simulink model "SdbTxSigProc"  (R2022b)
settings: Solver=FixedStepDiscrete  StartTime=0.0  StopTime=10.0  FixedStep=0.1  RelTol=1e-3
(part 1 of 4)
settings Model: Creator=blue.ge  LastModifiedBy=blue.ge
settings DataIOCC: SaveFormat=StructureWithTime
settings OptimizationCC: DefaultParameterBehavior=Inlined  EnhancedBackFolding=on  PassReuseOutputArgsAs=Individual arguments  ZeroExternalMemoryAtStartup=off  ZeroInternalMemoryAtStartup=off  OptimizeBlockOrder=speed  DifferentSizesBufferReuse=on  OptimizationCustomize=off  EfficientTunableParamExpr=on
settings DebuggingCC: ParameterTunabilityLossMsg=error
settings ModelReferenceCC: ModelReferenceNumInstancesAllowed=Single
settings SFSimCC: MATLABDynamicMemAlloc=off
settings RTWCC: Description=AUTOSAR  SystemTargetFile=autosar.tlc  GenCodeOnly=on  TemplateMakefile=ert_default_tmf
settings CodeAppCC: ForceParamTrailComments=on  IgnoreCustomStorageClasses=off  OperatorAnnotations=on  SimulinkDataObjDesc=on  SFDataObjDesc=on  InsertBlockDesc=on
settings STFCustomTargetCC: TargetLangStandard=C99 (ISO)  MultiwordLength=256  ParMdlRefBuildCompliant=on  CompOptLevelCompliant=on  IncludeMdlTerminateFcn=off  CombineOutputUpdateFcns=on  SuppressErrorStatus=on  ERTCustomFileBanners=on  SupportNonFinite=off  SupportComplex=off  SupportContinuousTime=off  SupportNonInlinedSFcns=off  RemoveResetFunc=on  MATLABClassNameForMDSCustomization=Simulink.SoftwareTarget.ERTCustomization  AutosarCompliant=on  UseToolchainInfoCompliant=on  LookupTableObjectStructAxisOrder=2,1,3,4,...  LUTObjectStructOrderEvenSpacing=Size,Table,Breakpoints  SystemTargetFile=autosar.tlc
settings ERTTargetCC: TargetLangStandard=C99 (ISO)  ConcurrentExecutionCompliant=off  IncludeMdlTerminateFcn=off  SuppressErrorStatus=on  SupportNonFinite=off  SupportComplex=off  ModelStepFunctionPrototypeControlCompliant=off  CPPClassGenCompliant=off  AutosarCompliant=on  LookupTableObjectStructAxisOrder=2,1,3,4,...  LUTObjectStructOrderEvenSpacing=Size,Table,Breakpoints  InlinedParameterPlacement=NonHierarchical  ERTCustomFileTemplate=example_file_process.tlc

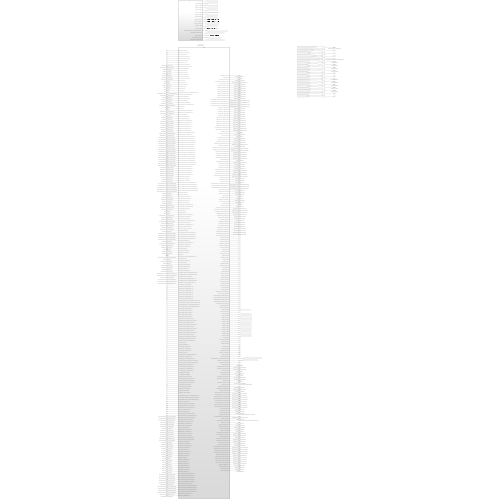
[thumbnail: Simulink's stored preview of the top-level diagram]
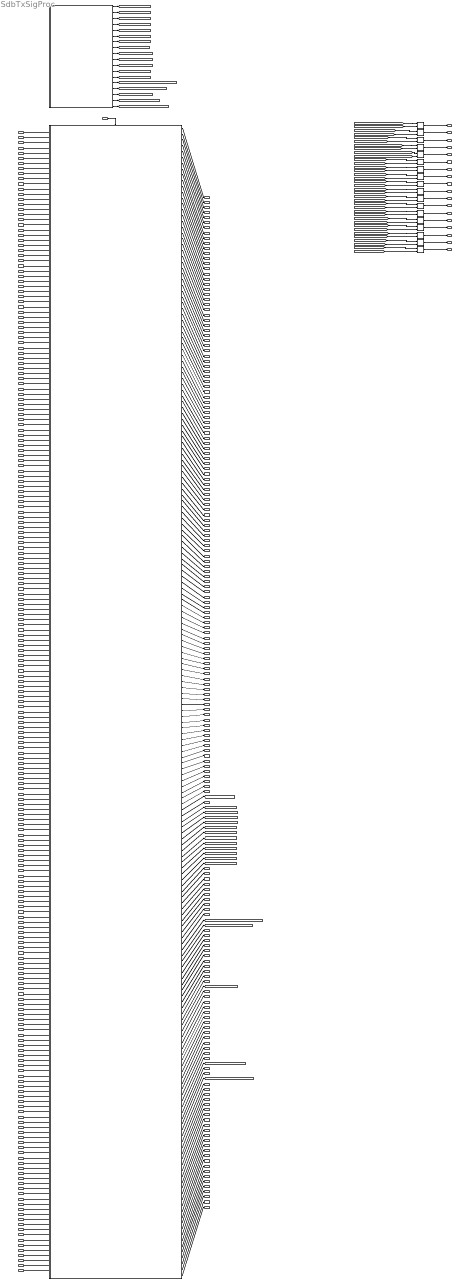
== system SdbTxSigProc ==
  SdbTxSigProc_Runnable : Inport  SampleTime=0.1  OutputFunctionCall=on
  AirTAftHvacDes_AirTAftHvacDes : Inport  Port=2  OutDataTypeStr=T30  PortDimensions=1
  AirTFbFromClima_Ngcc_AirTFbFromClima : Inport  Port=3  OutDataTypeStr=Bus: AirTFbFromClimaRec  PortDimensions=1  BusOutputAsStruct=on
  AmbTEstimd_Ngcc_AmbTEstimd : Inport  Port=4  OutDataTypeStr=Bus: AmbTEstimd  PortDimensions=1  BusOutputAsStruct=on
  AmbTFildForClimaNgcc_AmbTFildForClima : Inport  Port=5  OutDataTypeStr=T30  PortDimensions=1
  AmbTFildForClimaQf_AmbTFildForClimaQf : Inport  Port=6  OutDataTypeStr=Enum: GenQf1  PortDimensions=1
  CdsnProbltyToExt_CdsnProbltyToExt : Inport  Port=7  OutDataTypeStr=Fac30  PortDimensions=1
  ClimaActv_Ngcc_ClimaActv : Inport  Port=8  OutDataTypeStr=Enum: OnOff1  PortDimensions=1
  ClimaActvAlrmSts_Ngcc_ClimaActvAlrmSts : Inport  Port=9  OutDataTypeStr=Enum: OnOff1  PortDimensions=1
  ClimaCoolgPwrThermPred_ClimaCoolgPwrThermPred : Inport  Port=10  OutDataTypeStr=Bus: ClimaCoolgPwrThermPred  PortDimensions=1  BusOutputAsStruct=on
  ClimaDefrstMaxReq_ClimaDefrstMaxReq : Inport  Port=11  OutDataTypeStr=Boolean  PortDimensions=1
  ClimaOffReq_Ngcc_ClimaOffReq : Inport  Port=12  OutDataTypeStr=Enum: ClimaOffReq  PortDimensions=1
  ClimaPwrCns_Ngcc_ClimaPwrCns : Inport  Port=13  OutMin=0  OutMax=2047  OutDataTypeStr=Pwr30  PortDimensions=1
  ClimaPwrCnsPred_ClimaPwrCnsPred : Inport  Port=14  OutDataTypeStr=Bus: ClimaPwrCnsPredRec1  PortDimensions=1  BusOutputAsStruct=on
  ClimaPwrCritDes_Ngcc_ClimaPwrCritDes : Inport  Port=15  OutMin=0  OutMax=2047  OutDataTypeStr=Pwr30  PortDimensions=1
  ClimaPwrNormDes_Ngcc_ClimaPwrNormDes : Inport  Port=16  OutMin=0  OutMax=2047  OutDataTypeStr=Pwr30  PortDimensions=1
  ClimaStrtTi_ClimaStrtTi : Inport  Port=17  OutDataTypeStr=CarTiGlb  PortDimensions=1
  ClimaSts_Ngcc_ClimaSts : Inport  Port=18  OutDataTypeStr=Enum: ClimaSts  PortDimensions=1
  ClimaTiOfEnd_Ngcc_ClimaTiOfEnd : Inport  Port=19  OutDataTypeStr=CarTiGlb  PortDimensions=1
  ClimaTmrSts_ClimaTmrSts : Inport  Port=20  OutDataTypeStr=Enum: OnOff1  PortDimensions=1
  ClimaWarn_ClimaWarn : Inport  Port=21  OutDataTypeStr=Enum: ClimaWarn  PortDimensions=1
  ClngActv_ClngActv : Inport  Port=22  OutDataTypeStr=Enum: OnOffNoReq  PortDimensions=1
  CmptmtAirTEstimdAtRowFirstLoResl_Ngcc_CmptmtAirTEstimdAtRowFirstLoResl : Inport  Port=23  OutMin=-60  OutMax=125  OutDataTypeStr=TForCmptmt  PortDimensions=1
  CmptmtAirTEstimdExtd_Ngcc_CmptmtAirTEstimdExtd : Inport  Port=24  OutDataTypeStr=Bus: CmptmtAirTEstimdExtd  PortDimensions=1  BusOutputAsStruct=on
  CmptmtAirTFbExtd_CmptmtAirTFbExtd : Inport  Port=25  OutDataTypeStr=Bus: CmptmtAirTEstimdExtd  PortDimensions=1  BusOutputAsStruct=on
  CmptmtRelHumToExt_CmptmtRelHumToExt : Inport  Port=26  OutDataTypeStr=Bus: CmptmtRelHumToExt  PortDimensions=1  BusOutputAsStruct=on
  CoolgStsForDisp_CoolgStsForDisp : Inport  Port=27  OutDataTypeStr=Enum: CoolgStsForDisp  PortDimensions=1
  CupHoldrActvAllwd_Ngcc_CupHoldrActvAllwd : Inport  Port=28  OutDataTypeStr=ScrHeatrEgyPwrCns1  PortDimensions=1
  CustClimaOvrdNotifForHmiCpt_CustClimaOvrdNotifForHmiCpt : Inport  Port=29  OutDataTypeStr=Enum: Flg1  PortDimensions=1
  DefrstReq_DefrstReq : Inport  Port=30  OutDataTypeStr=Bus: DefrstReq  PortDimensions=1  BusOutputAsStruct=on
  DewVal_DewVal : Inport  Port=31  OutDataTypeStr=Bus: AirDewMeasValReq  PortDimensions=1  BusOutputAsStruct=on
  DrvrSeatHeatgPwrAllwd_Ngcc_DrvrSeatHeatgPwrAllwd : Inport  Port=32  OutDataTypeStr=UInt8  PortDimensions=1
  DrvrSeatVentnPwrAllwd_Ngcc_DrvrSeatVentnPwrAllwd : Inport  Port=33  OutDataTypeStr=UInt8  PortDimensions=1
  EcoClimasts_Ngcc_EcoClimasts : Inport  Port=34  OutDataTypeStr=Enum: OnOff1  PortDimensions=1
  EcoModForClimaActv_EcoModForClimaActv : Inport  Port=35  OutDataTypeStr=Enum: OnOff1  PortDimensions=1
  EgyDesForClima_Ngcc_EgyDesForClima : Inport  Port=36  OutDataTypeStr=Egy2  PortDimensions=1
  EngRunngReqByClima_Ngcc_EngRunngReqByClima : Inport  Port=37  OutDataTypeStr=Enum: EngRunngReq  PortDimensions=1
  EngSpdIncIdleForClima_Ngcc_EngSpdIncIdleForClima : Inport  Port=38  OutDataTypeStr=Enum: Flg1  PortDimensions=1
  EvaprTFrntFildForClima_EvaprTFrntFildForClima : Inport  Port=39  OutDataTypeStr=T30  PortDimensions=1
  EvaprTFrntFildQfForClima_EvaprTFrntFildQfForClima : Inport  Port=40  OutDataTypeStr=Boolean  PortDimensions=1
  HmiDefrstReqForClima_HmiDefrstReqForClima : Inport  Port=41  OutDataTypeStr=Enum: OnOff1  PortDimensions=1
  HvacAirMFlowEstimd_Ngcc_HvacAirMFlowEstimd : Inport  Port=42  OutMin=0  OutMax=1000  OutDataTypeStr=MFlow1  PortDimensions=1
  HvacAirMFlowEstimdInHeatElm_HvacAirMFlowEstimdInHeatElm : Inport  Port=43  OutDataTypeStr=AirFlow30  PortDimensions=1
  HvacAirMFlowReEstimd_Ngcc_HvacAirMFlowReEstimd : Inport  Port=44  OutMin=0  OutMax=1000  OutDataTypeStr=MFlow1  PortDimensions=1
  HvacAirTFildForRowFirstAtFlrLe_HvacAirTFildForRowFirstAtFlrLe : Inport  Port=45  OutDataTypeStr=T30  PortDimensions=1
  HvacAirTFildForRowFirstAtFlrRi_HvacAirTFildForRowFirstAtFlrRi : Inport  Port=46  OutDataTypeStr=T30  PortDimensions=1
  HvacAirTFildForRowFirstAtVentLe_HvacAirTFildForRowFirstAtVentLe : Inport  Port=47  OutDataTypeStr=T30  PortDimensions=1
  HvacAirTFildForRowFirstAtVentRi_HvacAirTFildForRowFirstAtVentRi : Inport  Port=48  OutDataTypeStr=T30  PortDimensions=1
  HvacAirTFildForRowSecAtFlrLe_HvacAirTFildForRowSecAtFlrLe : Inport  Port=49  OutDataTypeStr=T30  PortDimensions=1
  HvacAirTFildForRowSecAtFlrRi_HvacAirTFildForRowSecAtFlrRi : Inport  Port=50  OutDataTypeStr=T30  PortDimensions=1
  HvacAirTFildForRowSecAtVentLe_HvacAirTFildForRowSecAtVentLe : Inport  Port=51  OutDataTypeStr=T30  PortDimensions=1
  HvacAirTFildForRowSecAtVentRi_HvacAirTFildForRowSecAtVentRi : Inport  Port=52  OutDataTypeStr=T30  PortDimensions=1
  HvacAirTForRowFirstAtFlrLeRaw_HvacAirTForRowFirstAtFlrLeRaw : Inport  Port=53  OutDataTypeStr=Bus: HvacAirTForRowFirstAtFlrLe  PortDimensions=1  BusOutputAsStruct=on
  HvacAirTForRowFirstAtFlrRiRaw_HvacAirTForRowFirstAtFlrRiRaw : Inport  Port=54  OutDataTypeStr=Bus: HvacAirTForRowFirstAtFlrRi  PortDimensions=1  BusOutputAsStruct=on
  HvacAirTForRowFirstAtVentRiRaw_HvacAirTForRowFirstAtVentRiRaw : Inport  Port=55  OutDataTypeStr=Bus: HvacAirTForRowFirstAtVentRi  PortDimensions=1  BusOutputAsStruct=on
  HvacAirTForRowSecAtFlrLeRaw_HvacAirTForRowSecAtFlrLeRaw : Inport  Port=56  OutDataTypeStr=Bus: HvacAirTForRowSecAtFlrLe  PortDimensions=1  BusOutputAsStruct=on
  HvacAirTForRowSecAtFlrRiRaw_HvacAirTForRowSecAtFlrRiRaw : Inport  Port=57  OutDataTypeStr=Bus: HvacAirTForRowSecAtFlrRi  PortDimensions=1  BusOutputAsStruct=on
  HvacAirTForRowSecAtVentLeRaw_HvacAirTForRowSecAtVentLeRaw : Inport  Port=58  OutDataTypeStr=Bus: HvacAirTForRowSecAtVentLe  PortDimensions=1  BusOutputAsStruct=on
  HvacAirTForRowSecAtVentRiRaw_HvacAirTForRowSecAtVentRiRaw : Inport  Port=59  OutDataTypeStr=Bus: HvacAirTForRowSecAtVentRi  PortDimensions=1  BusOutputAsStruct=on
  HvacAirTReqAtRowFirstLe_HvacAirTReqAtRowFirstLe : Inport  Port=60  OutDataTypeStr=T30  PortDimensions=1
  HvacAirTReqAtRowFirstRi_HvacAirTReqAtRowFirstRi : Inport  Port=61  OutDataTypeStr=T30  PortDimensions=1
  HvacAirTReqAtRowSecLe_HvacAirTReqAtRowSecLe : Inport  Port=62  OutDataTypeStr=T30  PortDimensions=1
  HvacAirTReqAtRowSecRi_HvacAirTReqAtRowSecRi : Inport  Port=63  OutDataTypeStr=T30  PortDimensions=1
  HvacCondsorTspEvaprTFrnt_HvacCondsorTspEvaprTFrnt : Inport  Port=64  OutDataTypeStr=T30  PortDimensions=1
  HvacCoolgEnaFrnt_Ngcc_HvacCoolgEnaFrnt : Inport  Port=65  OutDataTypeStr=Enum: OnOff1  PortDimensions=1
  HvacCoolgEnaRe_Ngcc_HvacCoolgEnaRe : Inport  Port=66  OutDataTypeStr=Enum: OnOff1  PortDimensions=1
  HvacEvaprTSpFrnt_Ngcc_HvacEvaprTSpFrnt : Inport  Port=67  OutDataTypeStr=HvacEvaprTSp  PortDimensions=1
  HvacFlowFrntActForRowFirstAtVent_HvacFlowFrntActForRowFirstAtVent : Inport  Port=68  OutDataTypeStr=AirFlow30  PortDimensions=1
  HvacFlowFrntActForRowFirstAtVent_HvacFlowFrntActForRowFirstAtVentLe : Inport  Port=69  OutDataTypeStr=AirFlow30  PortDimensions=1
  HvacFlowFrntActForRowFirstAtVent_HvacFlowFrntActForRowFirstAtVentRi : Inport  Port=70  OutDataTypeStr=AirFlow30  PortDimensions=1
  HvacFlowFrntActForRowFirstAtVent_HvacFlowFrntActForRowFirstAtVentSideLe : Inport  Port=71  OutDataTypeStr=AirFlow30  PortDimensions=1
  HvacFlowFrntActForRowFirstAtVent_HvacFlowFrntActForRowFirstAtVentSideRi : Inport  Port=72  OutDataTypeStr=AirFlow30  PortDimensions=1
  HvacHeatgReqInt_HvacHeatgReqInt : Inport  Port=73  OutDataTypeStr=Enum: OnOff1  PortDimensions=1
  HvacRecircAct_Ngcc_HvacRecircAct : Inport  Port=74  OutDataTypeStr=Perc4  PortDimensions=1
  HvEgyDesForClima_Ngcc_HvEgyDesForClima : Inport  Port=75  OutDataTypeStr=HvEgyDesForClima  PortDimensions=1
  InPm25SnsrActnReq_Ngcc_InPm25SnsrActnReq : Inport  Port=76  OutDataTypeStr=Enum: OnOff1  PortDimensions=1
  IntPm25HiPopUp_Ngcc_IntPm25HiPopUp : Inport  Port=77  OutDataTypeStr=Enum: IntPmHiPopUp  PortDimensions=1
  LvlOfClimaCmft_Ngcc_LvlOfClimaCmft : Inport  Port=78  OutDataTypeStr=Enum: LvlOfClimaCmft  PortDimensions=1
  PassSeatHeatgPwrAllwd_Ngcc_PassSeatHeatgPwrAllwd : Inport  Port=79  OutDataTypeStr=UInt8  PortDimensions=1
  PassSeatVentnPwrAllwd_Ngcc_PassSeatVentnPwrAllwd : Inport  Port=80  OutDataTypeStr=UInt8  PortDimensions=1
  PostDrvgClimaAvl_Ngcc_PostDrvgClimaAvl : Inport  Port=81  OutDataTypeStr=Enum: OnOff1  PortDimensions=1
  PreClngActv_PreClngActv : Inport  Description=|  Port=82  OutDataTypeStr=Enum: OnOffNoReq  PortDimensions=1
      | The CCM shall request PM2.5 measurement during pre cleaning with the signal.
      | 0 = Signal value not valid (default)
      | 1= Pre Cleaning active
      | 2= No request
  PreClngNotif_PreClngNotif : Inport  Port=83  OutDataTypeStr=Enum: PreClngNotif  PortDimensions=1
  PwrAllwdForElecAirVentn_Ngcc_PwrAllwdForElecAirVentn : Inport  Port=84  OutDataTypeStr=ScrHeatrEgyPwrCns1  PortDimensions=1
  PwrAllwdForSeatHeatg_PwrAllwdForSeatHeatg : Inport  Port=85  OutDataTypeStr=Pwr33  PortDimensions=1
  PwrAllwdForSeatVent_PwrAllwdForSeatVent : Inport  Port=86  OutDataTypeStr=Pwr33  PortDimensions=1
  PwrChrgDetdForPrkgHeatrElec_PwrChrgDetdForPrkgHeatrElec : Inport  Port=87  OutDataTypeStr=Boolean  PortDimensions=1
  PwrCnsHeatgElecPred_PwrCnsHeatgElecPred : Inport  Port=88  OutDataTypeStr=Bus: PwrCnsHeatgElecPred  PortDimensions=1  BusOutputAsStruct=on
  PwrEstimdAtStbForClima_Ngcc_PwrEstimdAtStbForClima : Inport  Port=89  OutMin=0  OutMax=2047  OutDataTypeStr=Pwr30  PortDimensions=1
  RlyCmftForClimaReq_Ngcc_RlyCmftForClimaReq : Inport  Port=90  OutDataTypeStr=Enum: OnOff1  PortDimensions=1
  RlyCrashForClimaReq_Ngcc_RlyCrashForClimaReq : Inport  Port=91  OutDataTypeStr=Enum: OnOff1  PortDimensions=1
  SeatHeatgAutCdn_SeatHeatgAutCdn : Inport  Port=92  OutDataTypeStr=Enum: Flg1  PortDimensions=1
  SecRowLeSeatHeatgPwrAllwd_Ngcc_SecRowLeSeatHeatgPwrAllwd : Inport  Port=93  OutDataTypeStr=UInt8  PortDimensions=1
  SecRowLeSeatVentnPwrAllwd_Ngcc_SecRowLeSeatVentnPwrAllwd : Inport  Port=94  OutDataTypeStr=UInt8  PortDimensions=1
  SecRowRiSeatHeatgPwrAllwd_Ngcc_SecRowRiSeatHeatgPwrAllwd : Inport  Port=95  OutDataTypeStr=UInt8  PortDimensions=1
  SecRowRiSeatVentnPwrAllwd_Ngcc_SecRowRiSeatVentnPwrAllwd : Inport  Port=96  OutDataTypeStr=UInt8  PortDimensions=1
  SteerWhlHeatgAllwd_SteerWhlHeatgAllwd : Inport  Port=97  OutDataTypeStr=ClimaLoadPwr  PortDimensions=1
  SteerWhlHeatgDurgClima_Ngcc_SteerWhlHeatgDurgClima : Inport  Port=98  OutDataTypeStr=Boolean  PortDimensions=1
  UBoostReqByClima_Ngcc_UBoostReqByClima : Inport  Port=99  OutDataTypeStr=Enum: ReqSts1  PortDimensions=1
  VehMktForPmSnsr_Ngcc_VehMktForPmSnsr : Inport  Port=100  OutDataTypeStr=Bus: AirQlyMeasInValReq  PortDimensions=1  BusOutputAsStruct=on
  WindDewUppr_WindDewTUppr : Inport  Port=101  OutDataTypeStr=Bus: WindDewTUppr  PortDimensions=1  BusOutputAsStruct=on
  WindIceDetdFrnt_Ngcc_WindIceDetdFrnt : Inport  Port=102  OutDataTypeStr=Enum: OnOff1  PortDimensions=1
  WindIceDetdRe_Ngcc_WindIceDetdRe : Inport  Port=103  OutDataTypeStr=Enum: OnOff1  PortDimensions=1
  WindT_WindT : Inport  Port=104  OutDataTypeStr=Bus: WindT  PortDimensions=1  BusOutputAsStruct=on
  HvacAirTForRowFirstAtVentLeRaw_HvacAirTForRowFirstAtVentLeRaw : Inport  Port=105  OutDataTypeStr=Bus: HvacAirTForRowFirstAtVentLe  PortDimensions=1  BusOutputAsStruct=on
  ClimaStStrtUp_ClimaStStrtUp : Inport  Port=106  OutDataTypeStr=Enum: ClimaStStrtUp  PortDimensions=1
  AACAirClngActnNgcc_AACAirClngActnNgcc : Inport  Port=107  OutDataTypeStr=Enum: OnOff1  PortDimensions=1
  AirSrcReqNgcc_AirSrcReqNgcc : Inport  Port=108  OutDataTypeStr=Enum: OnOffNoReq  PortDimensions=1
  AirTAftHvacDesFirstLe_AirTAftHvacDesFirstLe : Inport  Port=109  OutDataTypeStr=T30  PortDimensions=1
  AirTAftHvacDesFirstRi_AirTAftHvacDesFirstRi : Inport  Port=110  OutDataTypeStr=T30  PortDimensions=1
  AirTAftHvacDesRe_AirTAftHvacDesRe : Inport  Port=111  OutDataTypeStr=T30  PortDimensions=1
  AirTAftHvacDesSecLe_AirTAftHvacDesSecLe : Inport  Port=112  OutDataTypeStr=T30  PortDimensions=1
  AirTFromHeatgEstimdFirstLeForCCM_AirTFromHeatgEstimdFirstLeForCCM : Inport  Port=113  OutDataTypeStr=T30  PortDimensions=1
  AirTFromHeatgEstimdFirstRiForCCM_AirTFromHeatgEstimdFirstRiForCCM : Inport  Port=114  OutDataTypeStr=T30  PortDimensions=1
  AirTFromHeatgEstimdForCCM_AirTFromHeatgEstimd : Inport  Port=115  OutDataTypeStr=T30  PortDimensions=1
  AirTFromHeatgEstimdReForCCM_AirTFromHeatgEstimdReForCCM : Inport  Port=116  OutDataTypeStr=T30  PortDimensions=1
  AirTFromHeatgEstimdSecLeForCCM_AirTFromHeatgEstimdSecLeForCCM : Inport  Port=117  OutDataTypeStr=T30  PortDimensions=1
  AutOxygenSplyStsFrmClimaNgcc_AutOxygenSplyStsFrmClimaNgcc : Inport  Port=118  OutDataTypeStr=Enum: OnOff1  PortDimensions=1
  CcmAACAirClngStsNgcc_CcmAACAirClngStsNgcc : Inport  Port=119  OutDataTypeStr=Enum: AACAirClngSts  PortDimensions=1
  CCMDataTraceZCUP1Ngcc_CCMDataTraceZCUP1Ngcc : Inport  Port=120  OutDataTypeStr=Bus: Develp1  PortDimensions=1  BusOutputAsStruct=on
  CCMDataTraceZCUP2Ngcc_CCMDataTraceZCUP2Ngcc : Inport  Port=121  OutDataTypeStr=Bus: Develp1  PortDimensions=1  BusOutputAsStruct=on
  CCMDataTraceZCUP3Ngcc_CCMDataTraceZCUP3Ngcc : Inport  Port=122  OutDataTypeStr=Bus: Develp1  PortDimensions=1  BusOutputAsStruct=on
  CCMDataTraceZCUP4Ngcc_CCMDataTraceZCUP4Ngcc : Inport  Port=123  OutDataTypeStr=Bus: Develp1  PortDimensions=1  BusOutputAsStruct=on
  CCMDataTraceZCUP5Ngcc_CCMDataTraceZCUP5Ngcc : Inport  Port=124  OutDataTypeStr=Bus: Develp1  PortDimensions=1  BusOutputAsStruct=on
  CCMDataTraceZCUP6Ngcc_CCMDataTraceZCUP6Ngcc : Inport  Port=125  OutDataTypeStr=Bus: Develp1  PortDimensions=1  BusOutputAsStruct=on
  CCMDataTraceZCUP7Ngcc_CCMDataTraceZCUP7Ngcc : Inport  Port=126  OutDataTypeStr=Bus: Develp1  PortDimensions=1  BusOutputAsStruct=on
  CcmElecAirDirCrtlReqSecCnsLePosXNgcc_CcmElecAirDirCrtlReqSecCnsLePosXNgcc : Inport  Port=127  OutDataTypeStr=UInt8  PortDimensions=1
  CcmElecAirDirCrtlReqSecCnsLePosYNgcc_CcmElecAirDirCrtlReqSecCnsLePosYNgcc : Inport  Port=128  OutDataTypeStr=UInt8  PortDimensions=1
  CcmElecAirDirCrtlReqSecCnsRiPosXNgcc_CcmElecAirDirCrtlReqSecCnsRiPosXNgcc : Inport  Port=129  OutDataTypeStr=UInt8  PortDimensions=1
  CcmElecAirDirCrtlReqSecCnsRiPosYNgcc_CcmElecAirDirCrtlReqSecCnsRiPosYNgcc : Inport  Port=130  OutDataTypeStr=UInt8  PortDimensions=1
  CCMFragCh1OvrdDyNgcc_CCMFragCh1OvrdDyNgcc : Inport  Port=131  OutDataTypeStr=Enum: Warn2  PortDimensions=1
  CCMFragCh2OvrdDyNgcc_CCMFragCh2OvrdDyNgcc : Inport  Port=132  OutDataTypeStr=Enum: Warn2  PortDimensions=1
  CCMFragCh3OvrdDyNgcc_CCMFragCh3OvrdDyNgcc : Inport  Port=133  OutDataTypeStr=Enum: Warn2  PortDimensions=1
  CCMFragCh4OvrdDyNgcc_CCMFragCh4OvrdDyNgcc : Inport  Port=134  OutDataTypeStr=Enum: Warn2  PortDimensions=1
  CCMFragCh5OvrdDyNgcc_CCMFragCh5OvrdDyNgcc : Inport  Port=135  OutDataTypeStr=Enum: Warn2  PortDimensions=1
  CCMFragraCh1AvlTiPrecNgcc_CCMFragraCh1AvlTiPrecNgcc : Inport  Port=136  OutMin=0  OutMax=100  OutDataTypeStr=Perc9  PortDimensions=1
  CCMFragraCh1AvlTiPrecNgcc_CCMFragraCh1AvlTiPrecNgcc_IsUpdated : Inport  Port=137  OutDataTypeStr=boolean  PortDimensions=1
  CCMFragraCh2AvlTiPrecNgcc_CCMFragraCh2AvlTiPrecNgcc : Inport  Port=138  OutMin=0  OutMax=100  OutDataTypeStr=Perc9  PortDimensions=1
  CCMFragraCh2AvlTiPrecNgcc_CCMFragraCh2AvlTiPrecNgcc_IsUpdated : Inport  Port=139  OutDataTypeStr=boolean  PortDimensions=1
  CCMFragraCh3AvlTiPrecNgcc_CCMFragraCh3AvlTiPrecNgcc : Inport  Port=140  OutMin=0  OutMax=100  OutDataTypeStr=Perc9  PortDimensions=1
  CCMFragraCh3AvlTiPrecNgcc_CCMFragraCh3AvlTiPrecNgcc_IsUpdated : Inport  Port=141  OutDataTypeStr=boolean  PortDimensions=1
  CCMFragraCh4AvlTiPrecNgcc_CCMFragraCh4AvlTiPrecNgcc : Inport  Port=142  OutMin=0  OutMax=100  OutDataTypeStr=Perc9  PortDimensions=1
  CCMFragraCh4AvlTiPrecNgcc_CCMFragraCh4AvlTiPrecNgcc_IsUpdated : Inport  Port=143  OutDataTypeStr=boolean  PortDimensions=1
  CCMFragraCh5AvlTiPrecNgcc_CCMFragraCh5AvlTiPrecNgcc : Inport  Port=144  OutMin=0  OutMax=100  OutDataTypeStr=Perc9  PortDimensions=1
  CCMFragraCh5AvlTiPrecNgcc_CCMFragraCh5AvlTiPrecNgcc_IsUpdated : Inport  Port=145  OutDataTypeStr=boolean  PortDimensions=1
  CcmWbmMasterReqLeLin1Ngcc_CcmWbmMasterReqLeLin1Ngcc : Inport  Port=146  OutDataTypeStr=UInt8  PortDimensions=1
  CcmWbmMasterReqRiLin1Ngcc_CcmWbmMasterReqRiLin1Ngcc : Inport  Port=147  OutDataTypeStr=UInt8  PortDimensions=1
  CdsnProblty_CdsnProblty : Inport  Port=148  OutDataTypeStr=Fac30  PortDimensions=1
  ChgFilElmRstNgcc_ChgFilElmRstNgcc : Inport  Port=149  OutMin=0  OutMax=3  OutDataTypeStr=UInt2  PortDimensions=1
  ClimaAtRowSecActvNgcc_ClimaAtRowSecActv : Inport  Port=150  OutDataTypeStr=Boolean  PortDimensions=1
  ClimaAtRowSecActvNgcc_ClimaAtRowSecActvLe : Inport  Port=151  OutDataTypeStr=Boolean  PortDimensions=1
  ClimaAtRowSecActvNgcc_ClimaAtRowSecActvRi : Inport  Port=152  OutDataTypeStr=Boolean  PortDimensions=1
  ClimaCtrlActvNgcc_ClimaCtrlActv : Inport  Port=153  OutDataTypeStr=Boolean  PortDimensions=1
  ClimaManModCmftPopUpReqNgcc_ClimaManModCmftPopUpReqNgcc : Inport  Port=154  OutDataTypeStr=Enum: PopUpReq  PortDimensions=1
  CmptmtAirDistbnRspNgcc_CmptmtAirDistbnRspNgcc : Inport  Port=155  OutDataTypeStr=Bus: CmptmtAirDistbnRsp  PortDimensions=1  BusOutputAsStruct=on
  CmptmtAirDistbnRspNgcc_CmptmtAirDistbnRspNgcc_IsUpdated : Inport  Port=156  OutDataTypeStr=boolean  PortDimensions=1
  CmptmtOxygenConcLvlFrmClimaNgcc_CmptmtOxygenConcLvlFrmClimaNgcc : Inport  Port=157  OutDataTypeStr=Enum: UnknowLvl  PortDimensions=1
  CmptmtOxygenConcVluFrmClimaNgcc_CmptmtOxygenConcVluFrmClimaNgcc : Inport  Port=158  OutMin=0  OutMax=100  OutDataTypeStr=Perc9  PortDimensions=1
  CoupldCtrlErrAtRowFirstLeNgcc_CoupldCtrlErrAtRowFirstLe : Inport  Port=159  OutDataTypeStr=T30  PortDimensions=1
  CoupldCtrlErrAtRowFirstRiNgcc_CoupldCtrlErrAtRowFirstRi : Inport  Port=160  OutDataTypeStr=T30  PortDimensions=1
  CoupldCtrlErrAtRowSecLeNgcc_CoupldCtrlErrAtRowSecLe : Inport  Port=161  OutDataTypeStr=T30  PortDimensions=1
  CoupldCtrlErrAtRowSecRiNgcc_CoupldCtrlErrAtRowSecRi : Inport  Port=162  OutDataTypeStr=T30  PortDimensions=1
  DutyCmdForMosBlw_DutyCmdForMosBlw : Inport  Port=163  OutMin=0  OutMax=100  OutDataTypeStr=Perc9  PortDimensions=1
  DutyCmdForPWMBlw_DutyCmdForPWMBlw : Inport  Port=164  OutMin=0  OutMax=100  OutDataTypeStr=Perc9  PortDimensions=1
  ExtVentToastFrmClima_Ngcc_ExtVentToastFrmClima : Inport  Port=165  OutDataTypeStr=Enum: ExtVentToastFrmClimaSts  PortDimensions=1
  FrntClimaManModCmftStsNgcc_FrntClimaManModCmftStsNgcc : Inport  Port=166  OutDataTypeStr=Enum: ClimaManModCmftSts  PortDimensions=1
  HighTempCleanRunningStsNgcc_HighTempCleanRunningStsNgcc : Inport  Port=167  OutDataTypeStr=Enum: OnOffNoReq  PortDimensions=1
  HighTempCleanSettingStsNgcc_HighTempCleanSettingStsNgcc : Inport  Port=168  OutDataTypeStr=Enum: OnOffNoReq  PortDimensions=1
  HvacAirTReAtFlrLeRaw_HvacAirTReAtFlrLeRaw : Inport  Port=169  OutDataTypeStr=Bus: HvacAirTReAtFlrLe  PortDimensions=1  BusOutputAsStruct=on
  HvacAirTReAtVentLeRaw_HvacAirTReAtVentLeRaw : Inport  Port=170  OutDataTypeStr=Bus: HvacAirTReAtVentLe  PortDimensions=1  BusOutputAsStruct=on
  HvacEvaprTSpReNgcc_HvacEvaprTSpReNgcc : Inport  Port=171  OutDataTypeStr=HvacEvaprTSp  PortDimensions=1
  HvacFanLvlFbNgcc_HvacFanLvlFbNgcc : Inport  Port=172  OutDataTypeStr=Enum: HmiHvacFanLvl  PortDimensions=1
  HvacFanLvlFbReNgcc_HvacFanLvlFbReNgcc : Inport  Port=173  OutDataTypeStr=Enum: HmiHvacFanLvl  PortDimensions=1
  HvacHeatElmAirMFlowEstimdFirstLeNgcc_HvacHeatElmAirMFlowEstimdFirstLeNgcc : Inport  Port=174  OutDataTypeStr=AirFlow30  PortDimensions=1
  HvacHeatElmAirMFlowEstimdFirstRiNgcc_HvacHeatElmAirMFlowEstimdFirstRiNgcc : Inport  Port=175  OutDataTypeStr=AirFlow30  PortDimensions=1
  HvacHeatElmAirMFlowEstimdSecLeNgcc_HvacHeatElmAirMFlowEstimdSecLeNgcc : Inport  Port=176  OutDataTypeStr=AirFlow30  PortDimensions=1
  HvacHeatgReqReInt_HvacHeatgReqReInt : Inport  Port=177  OutDataTypeStr=Enum: OnOff1  PortDimensions=1
  HvacHexAirTForRowFirstLeRaw_HvacHexAirTForRowFirstLeRaw : Inport  Port=178  OutDataTypeStr=Bus: HvacHexAirTForRowFirstLe  PortDimensions=1  BusOutputAsStruct=on
  HvacHexAirTForRowFirstRiRaw_HvacHexAirTForRowFirstRiRaw : Inport  Port=179  OutDataTypeStr=Bus: HvacHexAirTForRowFirstRi  PortDimensions=1  BusOutputAsStruct=on
  HvacHexAirTForRowSecLeRaw_HvacHexAirTForRowSecLeRaw : Inport  Port=180  OutDataTypeStr=Bus: HvacHexAirTForRowSecLe  PortDimensions=1  BusOutputAsStruct=on
  KeySynchrnztnLclNgcc_KeySynchrnztnLclNgcc : Inport  Port=181  OutDataTypeStr=Bus: RemSynchrnztnLcl  PortDimensions=1  BusOutputAsStruct=on
  OutlSovActvStsNgcc_OutlSovActvStsNgcc : Inport  Port=182  OutDataTypeStr=Enum: OnOff1  PortDimensions=1
  OxygenConcSnsrActnReqNgcc_OxygenConcSnsrActnReqNgcc : Inport  Port=183  OutDataTypeStr=Enum: OnOff1  PortDimensions=1
  OxygenConcSnsrStsFrmClimaNgcc_OxygenConcSnsrStsFrmClimaNgcc : Inport  Port=184  OutDataTypeStr=Enum: PmSnsrSts  PortDimensions=1
  OxygenSplyAvlStsFrmClimaNgcc_OxygenSplyAvlStsFrmClimaNgcc : Inport  Port=185  OutDataTypeStr=Enum: OxygenSplyAvlSts  PortDimensions=1
  OxygenSplyErrFrmClimaNgcc_OxygenSplyErrFrmClimaNgcc : Inport  Port=186  OutDataTypeStr=Enum: Err1  PortDimensions=1
  OxygenSplyPopUpReqNgcc_OxygenSplyPopUpReqNgcc : Inport  Port=187  OutDataTypeStr=Enum: OxygenSplyPopUpReq  PortDimensions=1
  OxygenSplyPwrAllwdNgcc_OxygenSplyPwrAllwdNgcc : Inport  Port=188  OutMin=0  OutMax=2047  OutDataTypeStr=Pwr30  PortDimensions=1
  OxygenSplyReqFaildNgcc_OxygenSplyReqFaildNgcc : Inport  Port=189  OutMin=0  OutMax=3  OutDataTypeStr=UInt2  PortDimensions=1
  OxygenSplyReqNgcc_OxygenSplyReqNgcc : Inport  Port=190  OutDataTypeStr=Enum: OxygenSplyReq  PortDimensions=1
  OxygenSplyVoiceFbNgcc_OxygenSplyVoiceFbNgcc : Inport  Port=191  OutDataTypeStr=Enum: OxygenSplyVoiceFb  PortDimensions=1
  PrkgClimaEngStReqNgcc_PrkgClimaEngStReqNgcc : Inport  Port=192  OutDataTypeStr=Enum: OnOff1  PortDimensions=1
  PwrCnsForHvacFanFrntNgcc_PwrCnsForHvacFanFrnt : Inport  Port=193  OutDataTypeStr=Pwr33  PortDimensions=1
  PwrCnsForHvacFanReNgcc_PwrCnsForHvacFanRe : Inport  Port=194  OutDataTypeStr=Pwr33  PortDimensions=1
  ReClimaManModCmftStsNgcc_ReClimaManModCmftStsNgcc : Inport  Port=195  OutDataTypeStr=Enum: ClimaManModCmftSts  PortDimensions=1
  RemOxygenSplyActvFaildNgcc_RemOxygenSplyActvFaildNgcc : Inport  Port=196  OutMin=0  OutMax=3  OutDataTypeStr=UInt2  PortDimensions=1
  TCSCmprStrtFltNgcc_TCSCmprStrtFltNgcc : Inport  Port=197  OutDataTypeStr=Enum: OnOff1  PortDimensions=1
  TCScoolgGrdReqNgcc_TCScoolgGrdReqNgcc : Inport  Port=198  OutMin=0  OutMax=15  OutDataTypeStr=UInt4  PortDimensions=1
  TCSCooltFlowReq1Ngcc_TCSCooltFlowReq1Ngcc : Inport  Port=199  OutDataTypeStr=CooltFlow1  PortDimensions=1
  TCSCooltTReq1Ngcc_TCSCooltTReq1Ngcc : Inport  Port=200  OutDataTypeStr=TempAtTime  PortDimensions=1
  TCSDoorStsNgcc_TCSDoorStsNgcc : Inport  Port=201  OutDataTypeStr=Enum: OnOff1  PortDimensions=1
  TCSDoorTopSts1Ngcc_TCSDoorTopSts1Ngcc : Inport  Port=202  OutDataTypeStr=Enum: WhlMotSysCluReq  PortDimensions=1
  TcsEcoModSts1Ngcc_TcsEcoModSts1Ngcc : Inport  Port=203  OutDataTypeStr=Enum: OnOff1  PortDimensions=1
  TcsFltNewSts1Ngcc_TcsFltNewSts1Ngcc : Inport  Port=204  OutDataTypeStr=Bus: Boolean32  PortDimensions=1  BusOutputAsStruct=on
  TCSFltStsNgcc_TCSFltStsNgcc : Inport  Port=205  OutMin=0  OutMax=15  OutDataTypeStr=UInt4  PortDimensions=1
  TCSModSwtStsNgcc_TCSModSwtStsNgcc : Inport  Port=206  OutDataTypeStr=Enum: TelmThermoTankReq  PortDimensions=1
  TCSnsrTStsNgcc_TCSnsrTStsNgcc : Inport  Port=207  OutDataTypeStr=TempAtTime  PortDimensions=1
  TCSRadrTreqNgcc_TCSRadrTreqNgcc : Inport  Port=208  OutDataTypeStr=TempAtTime  PortDimensions=1
  TCSRadrTStsNgcc_TCSRadrTStsNgcc : Inport  Port=209  OutDataTypeStr=TempAtTime  PortDimensions=1
  TCSTmrOverStsNgcc_TCSTmrOverStsNgcc : Inport  Port=210  OutMin=0  OutMax=31  OutDataTypeStr=TimeHr5  PortDimensions=1
  TCSTmrStrtStsNgcc_TCSTmrStrtStsNgcc : Inport  Port=211  OutDataTypeStr=Enum: OnOffNoReq  PortDimensions=1
  TCSTNowStsNgcc_TCSTNowStsNgcc : Inport  Port=212  OutDataTypeStr=TempAtTime  PortDimensions=1
  TgtHvacAirTForMaxTZoneNgcc_TgtHvacAirTForMaxTZoneNgcc : Inport  Port=213  OutDataTypeStr=T30  PortDimensions=1
  TgtHvacAirTForRowFirstLeNgcc_TgtHvacAirTForRowFirstLeNgcc : Inport  Port=214  OutMin=-256  OutMax=255.9  OutDataTypeStr=T2  PortDimensions=1
  TgtHvacAirTForRowFirstRiNgcc_TgtHvacAirTForRowFirstRiNgcc : Inport  Port=215  OutMin=-256  OutMax=255.9  OutDataTypeStr=T2  PortDimensions=1
  TgtHvacAirTForRowSecLeNgcc_TgtHvacAirTForRowSecLeNgcc : Inport  Port=216  OutMin=-256  OutMax=255.9  OutDataTypeStr=T2  PortDimensions=1
  TgtHvacAirTForRowSecRiNgcc_TgtHvacAirTForRowSecRiNgcc : Inport  Port=217  OutMin=-256  OutMax=255.9  OutDataTypeStr=T2  PortDimensions=1
  ThermoTSteDeoSts1Ngcc_ThermoTSteDeoSts1Ngcc : Inport  Port=218  OutDataTypeStr=UInt8  PortDimensions=1
  ThrdRowLeSeatHeatgPwrAllwdNgcc_ThrdRowLeSeatHeatgPwrAllwdNgcc : Inport  Port=219  OutDataTypeStr=UInt8  PortDimensions=1
  ThrdRowLeSeatVentnPwrAllwdNgcc_ThrdRowLeSeatVentnPwrAllwdNgcc : Inport  Port=220  OutDataTypeStr=UInt8  PortDimensions=1
  ThrdRowRiSeatHeatgPwrAllwdNgcc_ThrdRowRiSeatHeatgPwrAllwdNgcc : Inport  Port=221  OutDataTypeStr=UInt8  PortDimensions=1
  ThrdRowRiSeatVentnPwrAllwdNgcc_ThrdRowRiSeatVentnPwrAllwdNgcc : Inport  Port=222  OutDataTypeStr=UInt8  PortDimensions=1
  TiOfDeactvnForClimaCtrlNgcc_TiOfDeactvnForClimaCtrl : Inport  Port=223  OutDataTypeStr=Ti33  PortDimensions=1
  VMMChngNdMtnNgcc_VMMChngNdMtnNgcc : Inport  Port=224  OutDataTypeStr=Enum: OnOff1  PortDimensions=1
  AmbTEstimd_AmbTEstimd_merge : Merge
  CcmCaliSig10_CcmCaliSig10_merge : Merge
  CcmCaliSig11_CcmCaliSig11_merge : Merge
  CcmCaliSig12_CcmCaliSig12_merge : Merge
  CcmCaliSig1_CcmCaliSig1_merge : Merge
  CcmCaliSig2_CcmCaliSig2_merge : Merge
  CcmCaliSig3_CcmCaliSig3_merge : Merge
  CcmCaliSig4_CcmCaliSig4_merge : Merge
  CcmCaliSig5_CcmCaliSig5_merge : Merge
  CcmCaliSig6_CcmCaliSig6_merge : Merge
  CcmCaliSig7_CcmCaliSig7_merge : Merge
  CcmCaliSig8_CcmCaliSig8_merge : Merge
  CcmCaliSig9_CcmCaliSig9_merge : Merge
  CmptmtAirTEstimdAtRowFirstLoResl_CmptmtAirTEstimdAtRowFirstLoResl_merge : Merge
  CmptmtAirTEstimdExtd_CmptmtAirTEstimdExtd_merge : Merge
  HeatrAirTReq_HeatrAirTReq_merge : Merge
  HvacEvaprTSpFrnt_HvacEvaprTSpFrnt_merge : Merge
  HvacHeatrInletTempReq_HvacHeatrInletTempReq_merge : Merge
  SdbTxSigProc_Init : Subsystem
  SdbTxSigProc_Runnable_sys : Subsystem
  CcmCaliSig1_CcmCaliSig1 : Outport  OutDataTypeStr=Bus: UInt64Rec  PortDimensions=1  BusOutputAsStruct=on
  CcmCaliSig2_CcmCaliSig2 : Outport  Port=2  OutDataTypeStr=Bus: UInt64Rec  PortDimensions=1  BusOutputAsStruct=on
  CcmCaliSig3_CcmCaliSig3 : Outport  Port=3  OutDataTypeStr=Bus: UInt64Rec  PortDimensions=1  BusOutputAsStruct=on
  CcmCaliSig4_CcmCaliSig4 : Outport  Port=4  OutDataTypeStr=Bus: UInt64Rec  PortDimensions=1  BusOutputAsStruct=on
  CcmCaliSig5_CcmCaliSig5 : Outport  Port=5  OutDataTypeStr=Bus: UInt64Rec  PortDimensions=1  BusOutputAsStruct=on
  CcmCaliSig6_CcmCaliSig6 : Outport  Port=6  OutDataTypeStr=Bus: UInt64Rec  PortDimensions=1  BusOutputAsStruct=on
  CcmCaliSig7_CcmCaliSig7 : Outport  Port=7  OutDataTypeStr=Bus: UInt64Rec  PortDimensions=1  BusOutputAsStruct=on
  AmbTEstimd_AmbTEstimd : Outport  Port=8  OutDataTypeStr=Bus: AmbTEstimd  PortDimensions=1  BusOutputAsStruct=on
  CcmCaliSig10_CcmCaliSig10 : Outport  Port=9  OutDataTypeStr=Bus: UInt64Rec  PortDimensions=1  BusOutputAsStruct=on
  CcmCaliSig11_CcmCaliSig11 : Outport  Port=10  OutDataTypeStr=Bus: UInt64Rec  PortDimensions=1  BusOutputAsStruct=on
  CcmCaliSig12_CcmCaliSig12 : Outport  Port=11  OutDataTypeStr=Bus: UInt64Rec  PortDimensions=1  BusOutputAsStruct=on
  CcmCaliSig8_CcmCaliSig8 : Outport  Port=12  OutDataTypeStr=Bus: UInt64Rec  PortDimensions=1  BusOutputAsStruct=on
  CcmCaliSig9_CcmCaliSig9 : Outport  Port=13  OutDataTypeStr=Bus: UInt64Rec  PortDimensions=1  BusOutputAsStruct=on
  CmptmtAirTEstimdAtRowFirstLoResl_CmptmtAirTEstimdAtRowFirstLoResl : Outport  Port=14  OutMin=-60  OutMax=125  OutDataTypeStr=TForCmptmt  PortDimensions=1
  CmptmtAirTEstimdExtd_CmptmtAirTEstimdExtd : Outport  Port=15  OutDataTypeStr=Bus: CmptmtAirTEstimdExtd  PortDimensions=1  BusOutputAsStruct=on
  HeatrAirTReq_HeatrAirTReq : Outport  Port=16  OutMin=-60  OutMax=195  OutDataTypeStr=T4  PortDimensions=1
  HvacEvaprTSpFrnt_HvacEvaprTSpFrnt : Outport  Port=17  OutDataTypeStr=HvacEvaprTSp  PortDimensions=1
  HvacHeatrInletTempReq_HvacHeatrInletTempReq : Outport  Port=18  OutDataTypeStr=Bus: EvaprTFrnt  PortDimensions=1  BusOutputAsStruct=on
  AACAirClngActn_AACAirClngActn : Outport  Port=19  OutDataTypeStr=Enum: OnOff1  PortDimensions=1
  AirSrcReq_AirSrcReq : Outport  Port=20  OutDataTypeStr=Enum: OnOffNoReq  PortDimensions=1
  AmbTFildForClima_AmbTFildForClima : Outport  Port=21  OutDataTypeStr=AmbT  PortDimensions=1
  AutOxygenSplyStsFrmClima_AutOxygenSplyStsFrmClima : Outport  Port=22  OutDataTypeStr=Enum: OnOff1  PortDimensions=1
  CcmAACAirClngSts_CcmAACAirClngSts : Outport  Port=23  OutDataTypeStr=Enum: AACAirClngSts  PortDimensions=1
  CCMDataTraceZCUP1_CCMDataTraceZCUP1 : Outport  Port=24  OutDataTypeStr=Bus: Develp1  PortDimensions=1  BusOutputAsStruct=on
  CCMDataTraceZCUP2_CCMDataTraceZCUP2 : Outport  Port=25  OutDataTypeStr=Bus: Develp1  PortDimensions=1  BusOutputAsStruct=on
  CCMDataTraceZCUP3_CCMDataTraceZCUP3 : Outport  Port=26  OutDataTypeStr=Bus: Develp1  PortDimensions=1  BusOutputAsStruct=on
  CCMDataTraceZCUP4_CCMDataTraceZCUP4 : Outport  Port=27  OutDataTypeStr=Bus: Develp1  PortDimensions=1  BusOutputAsStruct=on
  CCMDataTraceZCUP5_CCMDataTraceZCUP5 : Outport  Port=28  OutDataTypeStr=Bus: Develp1  PortDimensions=1  BusOutputAsStruct=on
  CCMDataTraceZCUP6_CCMDataTraceZCUP6 : Outport  Port=29  OutDataTypeStr=Bus: Develp1  PortDimensions=1  BusOutputAsStruct=on
  CCMDataTraceZCUP7_CCMDataTraceZCUP7 : Outport  Port=30  OutDataTypeStr=Bus: Develp1  PortDimensions=1  BusOutputAsStruct=on
  CcmElecAirDirCrtlReqSecCnsLePosX_CcmElecAirDirCrtlReqSecCnsLePosX : Outport  Port=31  OutDataTypeStr=UInt8  PortDimensions=1
  CcmElecAirDirCrtlReqSecCnsLePosY_CcmElecAirDirCrtlReqSecCnsLePosY : Outport  Port=32  OutDataTypeStr=UInt8  PortDimensions=1
  CcmElecAirDirCrtlReqSecCnsRiPosX_CcmElecAirDirCrtlReqSecCnsRiPosX : Outport  Port=33  OutDataTypeStr=UInt8  PortDimensions=1
  CcmElecAirDirCrtlReqSecCnsRiPosY_CcmElecAirDirCrtlReqSecCnsRiPosY : Outport  Port=34  OutDataTypeStr=UInt8  PortDimensions=1
  CCMFragCh1OvrdDy_CCMFragCh1OvrdDy : Outport  Port=35  OutDataTypeStr=Enum: Warn2  PortDimensions=1
  CCMFragCh2OvrdDy_CCMFragCh2OvrdDy : Outport  Port=36  OutDataTypeStr=Enum: Warn2  PortDimensions=1
  CCMFragCh3OvrdDy_CCMFragCh3OvrdDy : Outport  Port=37  OutDataTypeStr=Enum: Warn2  PortDimensions=1
  CCMFragCh4OvrdDy_CCMFragCh4OvrdDy : Outport  Port=38  OutDataTypeStr=Enum: Warn2  PortDimensions=1
  CCMFragCh5OvrdDy_CCMFragCh5OvrdDy : Outport  Port=39  OutDataTypeStr=Enum: Warn2  PortDimensions=1
  CCMFragraCh1AvlTiPrec_CCMFragraCh1AvlTiPrec : Outport  Port=40  OutMin=0  OutMax=100  OutDataTypeStr=Perc9  PortDimensions=1
  CCMFragraCh2AvlTiPrec_CCMFragraCh2AvlTiPrec : Outport  Port=41  OutMin=0  OutMax=100  OutDataTypeStr=Perc9  PortDimensions=1
  CCMFragraCh3AvlTiPrec_CCMFragraCh3AvlTiPrec : Outport  Port=42  OutMin=0  OutMax=100  OutDataTypeStr=Perc9  PortDimensions=1
  CCMFragraCh4AvlTiPrec_CCMFragraCh4AvlTiPrec : Outport  Port=43  OutMin=0  OutMax=100  OutDataTypeStr=Perc9  PortDimensions=1
  CCMFragraCh5AvlTiPrec_CCMFragraCh5AvlTiPrec : Outport  Port=44  OutMin=0  OutMax=100  OutDataTypeStr=Perc9  PortDimensions=1
  CcmWbmMasterReqLeLin1_CcmWbmMasterReqLeLin1 : Outport  Port=45  OutDataTypeStr=UInt8  PortDimensions=1
  CcmWbmMasterReqRiLin1_CcmWbmMasterReqRiLin1 : Outport  Port=46  OutDataTypeStr=UInt8  PortDimensions=1
  ChgFilElmRst_ChgFilElmRst : Outport  Port=47  OutMin=0  OutMax=3  OutDataTypeStr=UInt2  PortDimensions=1
  ClimaAtRowSecActv_ClimaAtRowSecActv : Outport  Port=48  OutDataTypeStr=Boolean  PortDimensions=1
  ClimaCtrlActv_ClimaCtrlActv : Outport  Port=49  OutDataTypeStr=Boolean  PortDimensions=1
  ClimaCtrlTiOfDeactvn_ClimaCtrlTiOfDeactvn : Outport  Port=50  OutDataTypeStr=UInt16s  PortDimensions=1
  ClimaEstimdTForHeat_ClimaEstimdTForHeat : Outport  Port=51  OutMin=-256  OutMax=255.9  OutDataTypeStr=T2  PortDimensions=1
  ClimaEstimdTForReHeat_ClimaEstimdTForReHeat : Outport  Port=52  OutMin=-256  OutMax=255.9  OutDataTypeStr=T2  PortDimensions=1
  ClimaManModCmftPopUpReq_ClimaManModCmftPopUpReq : Outport  Port=53  OutDataTypeStr=Enum: PopUpReq  PortDimensions=1
  CmptmtAirDistbnRsp_CmptmtAirDistbnRsp : Outport  Port=54  OutDataTypeStr=Bus: CmptmtAirDistbnRsp  PortDimensions=1  BusOutputAsStruct=on
  CmptmtOxygenConcLvlFrmClima_CmptmtOxygenConcLvlFrmClima : Outport  Port=55  OutDataTypeStr=Enum: UnknowLvl  PortDimensions=1
  CmptmtOxygenConcVluFrmClima_CmptmtOxygenConcVluFrmClima : Outport  Port=56  OutMin=0  OutMax=100  OutDataTypeStr=Perc9  PortDimensions=1
  CoupldCtrlErrAtRowFirstLe_CoupldCtrlErrAtRowFirstLe : Outport  Port=57  OutMin=-256  OutMax=255.9  OutDataTypeStr=T2  PortDimensions=1
  CoupldCtrlErrAtRowFirstRi_CoupldCtrlErrAtRowFirstRi : Outport  Port=58  OutMin=-256  OutMax=255.9  OutDataTypeStr=T2  PortDimensions=1
  CoupldCtrlErrAtRowSecLe_CoupldCtrlErrAtRowSecLe : Outport  Port=59  OutMin=-256  OutMax=255.9  OutDataTypeStr=T2  PortDimensions=1
  CoupldCtrlErrAtRowSecRi_CoupldCtrlErrAtRowSecRi : Outport  Port=60  OutMin=-256  OutMax=255.9  OutDataTypeStr=T2  PortDimensions=1
  FrntBlwSpdReq_FrntBlwSpdReq : Outport  Port=61  OutDataTypeStr=BlowerSpd  PortDimensions=1
  FrntClimaManModCmftSts_FrntClimaManModCmftSts : Outport  Port=62  OutDataTypeStr=Enum: ClimaManModCmftSts  PortDimensions=1